simulink model "gate_V37_2016a_HIL_C"  (R2016a) (continued)
== system gate_V37_2016a_HIL_C/Gate/HardwareInputWrapper/MIL_Gate_C == (continued)
  Constant3/1 -> Bus Creator/10  "YellowLedMin"
  Constant5/1 -> Bus Creator/12  "GreenLedMin"
  Constant2/1 -> Bus Creator/9  "YellowLedMax"
  Signal Builder/11(BulbMax) -> Bus Creator/13  "BulbMax"
  Constant1/1 -> Bus Creator/8  "RedLedMin"
  Signal Builder/12(BulbMin) -> Bus Creator/14  "BulbMin"
  Constant6/1 -> Bus Creator/15  "BT_outputMax"
  Constant/1 -> Bus Creator/7  "RedLedMax"
  Signal Builder/10(MCMin) -> Bus Creator/6  "MCMin"
  Signal Builder/9(MCMax) -> Bus Creator/5  "MCMax"
  Signal Builder/8(MRMin) -> Bus Creator/4  "MRMin"
  Signal Builder/7(MRMax) -> Bus Creator/3  "MRMax"
  Signal Builder/6(MLMin) -> Bus Creator/2  "MLMin"
  Signal Builder/5(MLMax) -> Bus Creator/1  "MLMax"
  Constant7/1 -> Bus Creator/16  "BT_outputMin"
  Signal Builder/3(rightSpeedRaw) -> rightCurrentRaw/1
  Signal Builder/4(leftSpeedRaw) -> leftCurrentRaw/1

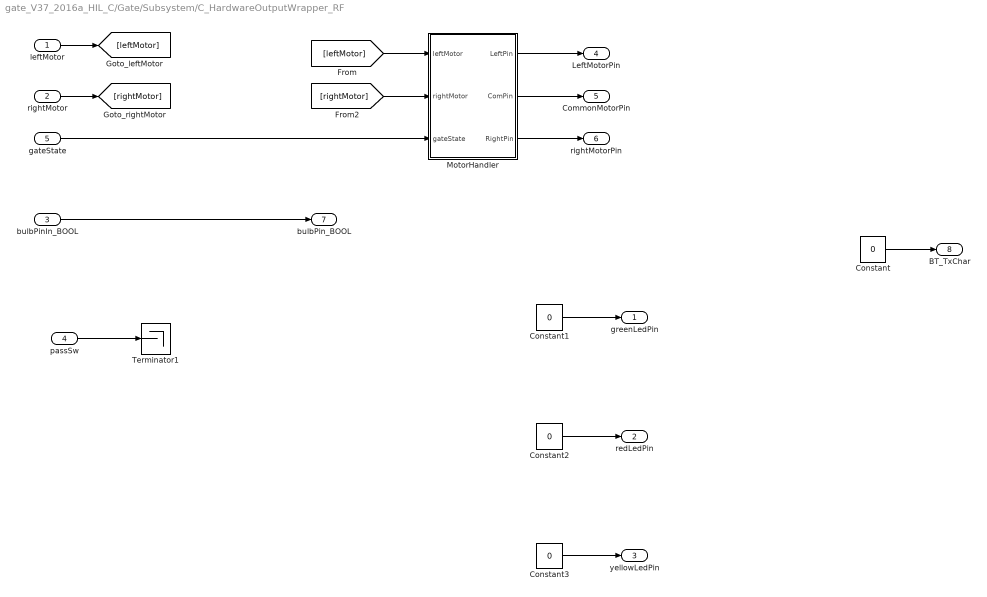
== system gate_V37_2016a_HIL_C/Gate/Subsystem/C_HardwareOutputWrapper_RF ==
  leftMotor : Inport
  rightMotor : Inport  Port=2
  bulbPinIn_BOOL : Inport  Port=3
  passSw : Inport  Port=4
  gateState : Inport  Port=5
  Constant{,1..3} (4 alike) : Constant  Value=0  SampleTime=-1
  MotorHandler : Subsystem
  Terminator1 : Terminator
  greenLedPin : Outport
  redLedPin : Outport  Port=2
  yellowLedPin : Outport  Port=3
  LeftMotorPin : Outport  Port=4
  CommonMotorPin : Outport  Port=5
  rightMotorPin : Outport  Port=6
  bulbPin_BOOL : Outport  Port=7
  BT_TxChar : Outport  Port=8
  wires:
  passSw/1 -> Terminator1/1
  Constant1/1 -> greenLedPin/1
  Constant2/1 -> redLedPin/1
  Constant3/1 -> yellowLedPin/1
  Constant/1 -> BT_TxChar/1
  rightMotor/1 -> [rightMotor]
  leftMotor/1 -> [leftMotor]
  bulbPinIn_BOOL/1 -> bulbPin_BOOL/1
  MotorHandler/3(RightPin) -> rightMotorPin/1
  MotorHandler/2(ComPin) -> CommonMotorPin/1
  MotorHandler/1(LeftPin) -> LeftMotorPin/1
  [rightMotor] -> MotorHandler/2(rightMotor)
  [leftMotor] -> MotorHandler/1(leftMotor)
  gateState/1 -> MotorHandler/3(gateState)

== system gate_V37_2016a_HIL_C/Gate/Subsystem/C_Variant_RF ==
  RFPressedOut_UINT8 : Inport
  passSwitch : Inport  Port=2
  current_sensor : Inport  Port=3
  CLOSED : Constant  Value=CLOSED  SampleTime=-1
  CLOSE_SIDE_VALUE : Constant  Value=CLOSE_SIDE_VALUE  SampleTime=-1
  CLOSING : Constant  Value=CLOSING  SampleTime=-1
  GateLogicStatemachine : Stateflow chart  TreatAsAtomicUnit=on
  INIT : Constant  Value=INIT  SampleTime=-1
  MOVING_TIMER : Constant  Value=MOVING_TIMER  SampleTime=-1
  OPEN : Constant  Value=OPEN  SampleTime=-1
  OPENING : Constant  Value=OPENING  SampleTime=-1
  OPENING_ACTIVE : Constant  Value=OPENING_ACTIVE  SampleTime=-1
  OPEN_SIDE_VALUE : Constant  Value=OPEN_SIDE_VALUE  SampleTime=-1
  STABLE_TIMER_VALUE : Constant  Value=STABLE_TIMER_VALUE  SampleTime=-1
  leftMotor : Outport
  rightMotor : Outport  Port=2
  bulbPin_BOOL : Outport  Port=3
  getState : Outport  Port=4
  wires:
  CLOSING/1 -> GateLogicStatemachine/9(CLOSING)
  OPENING/1 -> GateLogicStatemachine/7(OPENING)
  CLOSED/1 -> GateLogicStatemachine/8(CLOSED)
  OPEN/1 -> GateLogicStatemachine/10(OPEN)
  RFPressedOut_UINT8/1 -> GateLogicStatemachine/1(RFPressedOut_UINT8)
  GateLogicStatemachine/3(bulbPin_BOOL) -> bulbPin_BOOL/1
  current_sensor/1 -> GateLogicStatemachine/3(current_sensor)
  GateLogicStatemachine/1(leftMotor) -> leftMotor/1
  GateLogicStatemachine/2(rightMotor) -> rightMotor/1
  OPENING_ACTIVE/1 -> GateLogicStatemachine/4(OPENING_ACTIVE)
  OPEN_SIDE_VALUE/1 -> GateLogicStatemachine/5(OPEN_SIDE_VALUE)
  CLOSE_SIDE_VALUE/1 -> GateLogicStatemachine/6(CLOSE_SIDE_VALUE)
  passSwitch/1 -> GateLogicStatemachine/2(passSwitch)
  INIT/1 -> GateLogicStatemachine/11(INIT)
  MOVING_TIMER/1 -> GateLogicStatemachine/12(MOVING_TIMER)
  STABLE_TIMER_VALUE/1 -> GateLogicStatemachine/13(STABLE_TIMER_VALUE)
  GateLogicStatemachine/4(gateState) -> getState/1

== system gate_V37_2016a_HIL_C/Gate/Subsystem/CurrentFilter ==
  rightCurrentRaw : Inport
  leftCurrentRaw : Inport  Port=2
  10msSmapler : Stateflow chart  Commented=on  TreatAsAtomicUnit=on
  Subsystem : Subsystem
  current : Outport
  wires:
  leftCurrentRaw/1 -> Subsystem/2(leftCurrentRaw)
  rightCurrentRaw/1 -> Subsystem/1(rightCurrentRaw)
  Subsystem/1(current) -> current/1

== system gate_V37_2016a_HIL_C/Gate/outputPinsBlock/HIL_B ==
  greenLedPin : Inport
  redLedPin : Inport  Port=2
  yellowLed : Inport  Port=3
  leftMotorPin : Inport  Port=4
  commonMotorPin : Inport  Port=5
  rightMotorPin : Inport  Port=6
  bulbPin : Inport  Port=7
  Digital Output : arduinolib/Digital Output  pinNumber=11  blockPlatform=arduino
  Digital Output1 : arduinolib/Digital Output  pinNumber=12  blockPlatform=arduino
  Digital Output2 : arduinolib/Digital Output  pinNumber=13  blockPlatform=arduino
  S-Function Builder1 : S-Function  InitFcn=try, set_param(gcb,'FunctionName','A2PIN_Lib'), end  FunctionName=A2PIN_Lib  SFunctionModules=A2PIN_Lib_wrapper  SFunctionDeploymentMode=off  EnableBusSupport=off  mask(S-Function Builder){}
      mask help: Simulink:masks:SFunctionBuilder_MD
      mask init: same as gate_V37_2016a_HIL_C/Gate/HardwareInputWrapper/HIL_B/S-Function Builder1
      settings: CopyFcn=set_param(gcbh,'LinkStatus','none')  PreSaveFcn=try, set_param(gcb,'FunctionName','A2PIN_Lib'), end  OpenFcn=sfunctionwizard(gcbh)
      mask Display: plot(val(:,1),val(:,2)),disp(sys), port_label('input',1,'input')
  S-Function Builder2 : S-Function  InitFcn=try, set_param(gcb,'FunctionName','A3PIN_Lib'), end  FunctionName=A3PIN_Lib  SFunctionModules=A3PIN_Lib_wrapper  SFunctionDeploymentMode=off  EnableBusSupport=off  mask(S-Function Builder){}
      mask help: Simulink:masks:SFunctionBuilder_MD
      mask init: same as gate_V37_2016a_HIL_C/Gate/HardwareInputWrapper/HIL_B/S-Function Builder1
      settings: CopyFcn=set_param(gcbh,'LinkStatus','none')  PreSaveFcn=try, set_param(gcb,'FunctionName','A3PIN_Lib'), end  OpenFcn=sfunctionwizard(gcbh)
      mask Display: plot(val(:,1),val(:,2)),disp(sys), port_label('input',1,'input')
  S-Function Builder3 : S-Function  InitFcn=try, set_param(gcb,'FunctionName','A4PIN_Lib'), end  FunctionName=A4PIN_Lib  SFunctionModules=A4PIN_Lib_wrapper  SFunctionDeploymentMode=off  EnableBusSupport=off  mask(S-Function Builder){}
      mask help: Simulink:masks:SFunctionBuilder_MD
      mask init: same as gate_V37_2016a_HIL_C/Gate/HardwareInputWrapper/HIL_B/S-Function Builder1
      settings: CopyFcn=set_param(gcbh,'LinkStatus','none')  PreSaveFcn=try, set_param(gcb,'FunctionName','A4PIN_Lib'), end  OpenFcn=sfunctionwizard(gcbh)
      mask Display: plot(val(:,1),val(:,2)),disp(sys), port_label('input',1,'input')
  S-Function Builder4 : S-Function  InitFcn=try, set_param(gcb,'FunctionName','A5PIN_Lib'), end  FunctionName=A5PIN_Lib  SFunctionModules=A5PIN_Lib_wrapper  SFunctionDeploymentMode=off  EnableBusSupport=off  mask(S-Function Builder){}
      mask help: Simulink:masks:SFunctionBuilder_MD
      mask init: same as gate_V37_2016a_HIL_C/Gate/HardwareInputWrapper/HIL_B/S-Function Builder1
      settings: CopyFcn=set_param(gcbh,'LinkStatus','none')  PreSaveFcn=try, set_param(gcb,'FunctionName','A5PIN_Lib'), end  OpenFcn=sfunctionwizard(gcbh)
      mask Display: plot(val(:,1),val(:,2)),disp(sys), port_label('input',1,'input')
  wires:
  yellowLed/1 -> Digital Output2/1
  redLedPin/1 -> Digital Output/1
  greenLedPin/1 -> Digital Output1/1
  commonMotorPin/1 -> S-Function Builder3/1
  leftMotorPin/1 -> S-Function Builder2/1
  rightMotorPin/1 -> S-Function Builder4/1
  bulbPin/1 -> S-Function Builder1/1

== system gate_V37_2016a_HIL_C/Gate/outputPinsBlock/HIL_C ==
  leftMotorPin : Inport
  commonMotorPin : Inport  Port=2
  rightMotorPin : Inport  Port=3
  bulbPin : Inport  Port=4
  S-Function Builder1 : S-Function  InitFcn=try, set_param(gcb,'FunctionName','A2PIN_Lib'), end  FunctionName=A2PIN_Lib  SFunctionModules=A2PIN_Lib_wrapper  SFunctionDeploymentMode=off  EnableBusSupport=off  mask(S-Function Builder){}
      mask help: Simulink:masks:SFunctionBuilder_MD
      mask init: same as gate_V37_2016a_HIL_C/Gate/HardwareInputWrapper/HIL_B/S-Function Builder1
      settings: CopyFcn=set_param(gcbh,'LinkStatus','none')  PreSaveFcn=try, set_param(gcb,'FunctionName','A2PIN_Lib'), end  OpenFcn=sfunctionwizard(gcbh)
      mask Display: plot(val(:,1),val(:,2)),disp(sys), port_label('input',1,'input')
  S-Function Builder2 : S-Function  InitFcn=try, set_param(gcb,'FunctionName','A3PIN_Lib'), end  FunctionName=A3PIN_Lib  SFunctionModules=A3PIN_Lib_wrapper  SFunctionDeploymentMode=off  EnableBusSupport=off  mask(S-Function Builder){}
      mask help: Simulink:masks:SFunctionBuilder_MD
      mask init: same as gate_V37_2016a_HIL_C/Gate/HardwareInputWrapper/HIL_B/S-Function Builder1
      settings: CopyFcn=set_param(gcbh,'LinkStatus','none')  PreSaveFcn=try, set_param(gcb,'FunctionName','A3PIN_Lib'), end  OpenFcn=sfunctionwizard(gcbh)
      mask Display: plot(val(:,1),val(:,2)),disp(sys), port_label('input',1,'input')
  S-Function Builder3 : S-Function  InitFcn=try, set_param(gcb,'FunctionName','A4PIN_Lib'), end  FunctionName=A4PIN_Lib  SFunctionModules=A4PIN_Lib_wrapper  SFunctionDeploymentMode=off  EnableBusSupport=off  mask(S-Function Builder){}
      mask help: Simulink:masks:SFunctionBuilder_MD
      mask init: same as gate_V37_2016a_HIL_C/Gate/HardwareInputWrapper/HIL_B/S-Function Builder1
      settings: CopyFcn=set_param(gcbh,'LinkStatus','none')  PreSaveFcn=try, set_param(gcb,'FunctionName','A4PIN_Lib'), end  OpenFcn=sfunctionwizard(gcbh)
      mask Display: plot(val(:,1),val(:,2)),disp(sys), port_label('input',1,'input')
  S-Function Builder4 : S-Function  InitFcn=try, set_param(gcb,'FunctionName','A5PIN_Lib'), end  FunctionName=A5PIN_Lib  SFunctionModules=A5PIN_Lib_wrapper  SFunctionDeploymentMode=off  EnableBusSupport=off  mask(S-Function Builder){}
      mask help: Simulink:masks:SFunctionBuilder_MD
      mask init: same as gate_V37_2016a_HIL_C/Gate/HardwareInputWrapper/HIL_B/S-Function Builder1
      settings: CopyFcn=set_param(gcbh,'LinkStatus','none')  PreSaveFcn=try, set_param(gcb,'FunctionName','A5PIN_Lib'), end  OpenFcn=sfunctionwizard(gcbh)
      mask Display: plot(val(:,1),val(:,2)),disp(sys), port_label('input',1,'input')
  wires:
  bulbPin/1 -> S-Function Builder1/1
  rightMotorPin/1 -> S-Function Builder4/1
  leftMotorPin/1 -> S-Function Builder2/1
  commonMotorPin/1 -> S-Function Builder3/1

== system gate_V37_2016a_HIL_C/Gate/outputPinsBlock/MIL ==
  "leftMotorPin " : Inport
  "commonMotorPin " : Inport  Port=2
  "rightMotorPin " : Inport  Port=3
  greenLedPin : Inport  Port=4
  redLedPin : Inport  Port=5
  yellowLed : Inport  Port=6
  bulbPin : Inport  Port=7
  BT_TxChar : Inport  Port=8
  SimuOutputLimits : Inport  Port=9
  ScopeBlocks : Subsystem  VariantControl=VisualOutputs
  "VerificationBlocks " : Subsystem  VariantControl=VVOutputs

== system gate_V37_2016a_HIL_C/Gate/HardwareInputWrapper/HIL_B/BluetoothWrapper ==
  S-Function Builder : S-Function  InitFcn=try, set_param(gcb,'FunctionName','BluetoothLib'), end  FunctionName=BluetoothLib  SFunctionModules=BluetoothLib_wrapper  SFunctionDeploymentMode=off  EnableBusSupport=off  mask(S-Function Builder){}
      mask help: Simulink:masks:SFunctionBuilder_MD
      mask init: same as gate_V37_2016a_HIL_C/Gate/HardwareInputWrapper/HIL_B/S-Function Builder1
      settings: CopyFcn=set_param(gcbh,'LinkStatus','none')  PreSaveFcn=try, set_param(gcb,'FunctionName','BluetoothLib'), end  OpenFcn=sfunctionwizard(gcbh)
      mask Display: plot(val(:,1),val(:,2)),disp(sys), port_label('output',1,'BtCharRecieved')
  S-Function Builder1 : S-Function  Commented=on  InitFcn=try, set_param(gcb,'FunctionName','printingBlock'), end  FunctionName=printingBlock  SFunctionModules=printingBlock_wrapper  SFunctionDeploymentMode=off  EnableBusSupport=off  mask(S-Function Builder){}
      mask help: Simulink:masks:SFunctionBuilder_MD
      mask init: same as gate_V37_2016a_HIL_C/Gate/HardwareInputWrapper/HIL_B/S-Function Builder1
      settings: CopyFcn=set_param(gcbh,'LinkStatus','none')  PreSaveFcn=try, set_param(gcb,'FunctionName','printingBlock'), end  OpenFcn=sfunctionwizard(gcbh)
      mask Display: plot(val(:,1),val(:,2)),disp(sys), port_label('input',1,'u0'), port_label('output',1,'y0')
  Unit Delay : UnitDelay  Commented=on  SampleTime=-1
  bluetoothCharPressed : Outport
  wires:
  S-Function Builder/1 -> S-Function Builder1/1, Unit Delay/1, bluetoothCharPressed/1
  Unit Delay/1 -> ?

== system gate_V37_2016a_HIL_C/Gate/HardwareInputWrapper/HIL_B/ButtonsPressed ==
  S-Function Builder1 : S-Function  InitFcn=try, set_param(gcb,'FunctionName','Button_Lib'), end  FunctionName=Button_Lib  SFunctionModules=Button_Lib_wrapper  SFunctionDeploymentMode=off  EnableBusSupport=off  mask(S-Function Builder){}
      mask help: Simulink:masks:SFunctionBuilder_MD
      mask init: same as gate_V37_2016a_HIL_C/Gate/HardwareInputWrapper/HIL_B/S-Function Builder1
      settings: CopyFcn=set_param(gcbh,'LinkStatus','none')  PreSaveFcn=try, set_param(gcb,'FunctionName','Button_Lib'), end  OpenFcn=sfunctionwizard(gcbh)
      mask Display: plot(val(:,1),val(:,2)),disp(sys), port_label('output',1,'RFInput'), port_label('output',2,'passSw')
  RFPressed : Outport
  SWPressed : Outport  Port=2
  wires:
  S-Function Builder1/1 -> RFPressed/1
  S-Function Builder1/2 -> SWPressed/1

== system gate_V37_2016a_HIL_C/Gate/HardwareInputWrapper/HIL_B/EEPROM ==
  passSw : Inport
  passAssigned : Inport  Port=2
  passwordIn : Inport  Port=3
  EEPROM_Control : Stateflow chart  TreatAsAtomicUnit=on
  EEPROM_UPDATE : Subsystem  TreatAsAtomicUnit=on
  Terminator : Terminator
  Unit Delay : UnitDelay  SampleTime=-1
  Unit Delay1 : UnitDelay  SampleTime=-1
  passwordOut : Outport
  passAssignedOut : Outport  Port=2
  wires:
  EEPROM_UPDATE/3(clearComplete) -> Terminator/1
  EEPROM_UPDATE/5(passAssignedOut) -> passAssignedOut/1
  EEPROM_UPDATE/4(passwordOut) -> passwordOut/1
  EEPROM_UPDATE/2(writeComplete) -> [writeComplete]
  [writeComplete] -> Unit Delay1/1
  [readComplete] -> Unit Delay/1
  EEPROM_UPDATE/1(readComplete) -> [readComplete]
  passAssigned/1 -> [passAssigned]
  [passAssigned] -> EEPROM_UPDATE/5(passAssignedIn)
  [passAssigned] -> EEPROM_Control/2(passAssigned)
  passwordIn/1 -> EEPROM_UPDATE/4(passwordIn)
  EEPROM_Control/3(writeReq) -> EEPROM_UPDATE/3(writeReq)
  EEPROM_Control/2(readReq) -> EEPROM_UPDATE/2(readReq)
  EEPROM_Control/1(clearReq) -> EEPROM_UPDATE/1(clearReq)
  Unit Delay1/1 -> EEPROM_Control/4(writeComplete)
  Unit Delay/1 -> EEPROM_Control/3(readComplete)
  passSw/1 -> EEPROM_Control/1(passSw)

== system gate_V37_2016a_HIL_C/Gate/HardwareInputWrapper/HIL_B/KeypadWrapper ==
  KeypadLib : S-Function  InitFcn=try, set_param(gcb,'FunctionName','keypadLib'), end  FunctionName=keypadLib  SFunctionModules=keypadLib_wrapper  SFunctionDeploymentMode=off  EnableBusSupport=off  mask(S-Function Builder){}
      mask help: Simulink:masks:SFunctionBuilder_MD
      mask init: same as gate_V37_2016a_HIL_C/Gate/HardwareInputWrapper/HIL_B/S-Function Builder1
      settings: CopyFcn=set_param(gcbh,'LinkStatus','none')  PreSaveFcn=try, set_param(gcb,'FunctionName','keypadLib'), end  OpenFcn=sfunctionwizard(gcbh)
      mask Display: plot(val(:,1),val(:,2)),disp(sys), port_label('output',1,'charPressed')
  charPressed : Outport
  wires:
  KeypadLib/1 -> charPressed/1

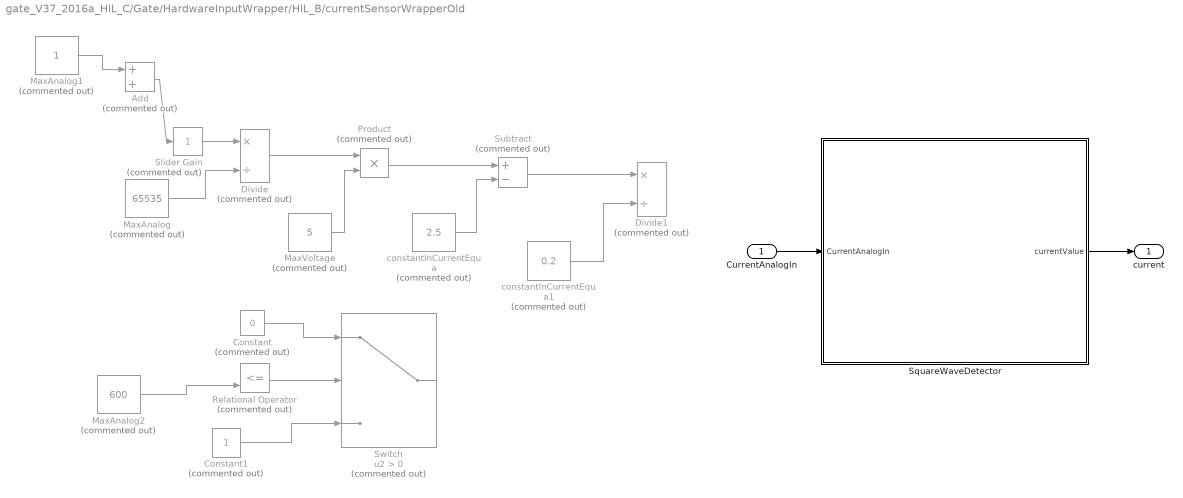
== system gate_V37_2016a_HIL_C/Gate/HardwareInputWrapper/HIL_B/currentSensorWrapperOld ==
  CurrentAnalogIn : Inport
  Add : Sum  Commented=on
  Constant : Constant  Commented=on  Value=0
  Constant1 : Constant  Commented=on
  Divide : Product  Commented=on  Inputs=*/
  Divide1 : Product  Commented=on  Inputs=*/
  MaxAnalog : Constant  Commented=on  Value=65535
  MaxAnalog1 : Constant  Commented=on
  MaxAnalog2 : Constant  Commented=on  Value=600
  MaxVoltage : Constant  Commented=on  Value=5
  Product : Product  Commented=on
  Relational Operator : RelationalOperator  Commented=on  Operator=<=  OutDataTypeStr=boolean
  Slider Gain : simulink/Math Operations/Slider Gain  Commented=on  low=0  gain=1  high=255
  SquareWaveDetector : Stateflow chart  TreatAsAtomicUnit=on
  Subtract : Sum  Commented=on  Inputs=+-
  Switch : Switch  Commented=on  Criteria=u2 > Threshold
  constantInCurrentEqua : Constant  Commented=on  Value=2.5
  constantInCurrentEqua1 : Constant  Commented=on  Value=0.2
  current : Outport
  wires:
  MaxAnalog/1 -> Divide/2
  Divide/1 -> Product/1
  MaxVoltage/1 -> Product/2
  Product/1 -> Subtract/1
  constantInCurrentEqua/1 -> Subtract/2
  Subtract/1 -> Divide1/1
  constantInCurrentEqua1/1 -> Divide1/2
  Slider Gain/1 -> Divide/1
  Add/1 -> Slider Gain/1
  MaxAnalog1/1 -> Add/1
  MaxAnalog2/1 -> Relational Operator/2
  Relational Operator/1 -> Switch/2
  Constant1/1 -> Switch/3
  Constant/1 -> Switch/1
  CurrentAnalogIn/1 -> SquareWaveDetector/1(CurrentAnalogIn)
  SquareWaveDetector/1(currentValue) -> current/1

== system gate_V37_2016a_HIL_C/Gate/HardwareInputWrapper/HIL_C/ButtonsPressed ==
  S-Function Builder1 : S-Function  InitFcn=try, set_param(gcb,'FunctionName','Button_Lib'), end  FunctionName=Button_Lib  SFunctionModules=Button_Lib_wrapper  SFunctionDeploymentMode=off  EnableBusSupport=off  mask(S-Function Builder){}
      mask help: Simulink:masks:SFunctionBuilder_MD
      mask init: same as gate_V37_2016a_HIL_C/Gate/HardwareInputWrapper/HIL_B/S-Function Builder1
      settings: CopyFcn=set_param(gcbh,'LinkStatus','none')  PreSaveFcn=try, set_param(gcb,'FunctionName','Button_Lib'), end  OpenFcn=sfunctionwizard(gcbh)
      mask Display: plot(val(:,1),val(:,2)),disp(sys), port_label('output',1,'RFInput'), port_label('output',2,'passSw')
  RFPressed : Outport
  SWPressed : Outport  Port=2
  wires:
  S-Function Builder1/1 -> RFPressed/1
  S-Function Builder1/2 -> SWPressed/1

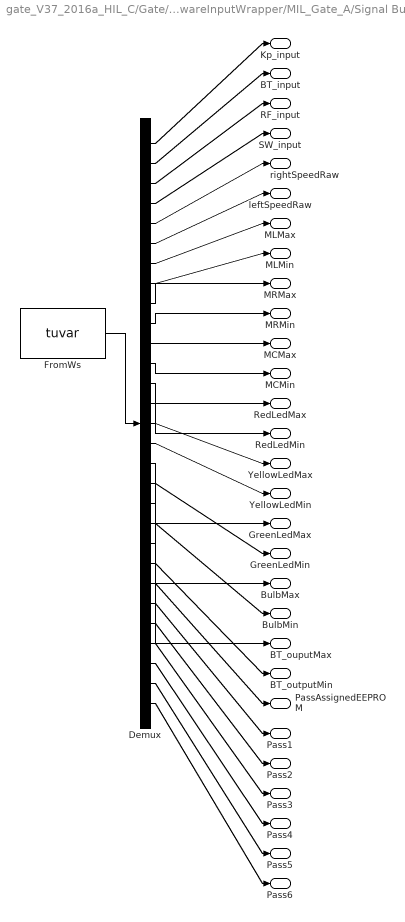
== system gate_V37_2016a_HIL_C/Gate/HardwareInputWrapper/MIL_Gate_A/Signal Builder ==
  Demux : Demux  Tag=STV Demux  Outputs=29
  FromWs : FromWorkspace  Tag=STV FromWs  VariableName=tuvar  SampleTime=0
  Kp_input : Outport  Tag=STV Outport
  BT_input : Outport  Tag=STV Outport  Port=2
  RF_input : Outport  Tag=STV Outport  Port=3
  SW_input : Outport  Tag=STV Outport  Port=4
  rightSpeedRaw : Outport  Tag=STV Outport  Port=5
  leftSpeedRaw : Outport  Tag=STV Outport  Port=6
  MLMax : Outport  Tag=STV Outport  Port=7
  MLMin : Outport  Tag=STV Outport  Port=8
  MRMax : Outport  Tag=STV Outport  Port=9
  MRMin : Outport  Tag=STV Outport  Port=10
  MCMax : Outport  Tag=STV Outport  Port=11
  MCMin : Outport  Tag=STV Outport  Port=12
  RedLedMax : Outport  Tag=STV Outport  Port=13
  RedLedMin : Outport  Tag=STV Outport  Port=14
  YellowLedMax : Outport  Tag=STV Outport  Port=15
  YellowLedMin : Outport  Tag=STV Outport  Port=16
  GreenLedMax : Outport  Tag=STV Outport  Port=17
  GreenLedMin : Outport  Tag=STV Outport  Port=18
  BulbMax : Outport  Tag=STV Outport  Port=19
  BulbMin : Outport  Tag=STV Outport  Port=20
  BT_ouputMax : Outport  Tag=STV Outport  Port=21
  BT_outputMin : Outport  Tag=STV Outport  Port=22
  PassAssignedEEPROM : Outport  Tag=STV Outport  Port=23
  Pass1 : Outport  Tag=STV Outport  Port=24
  Pass2 : Outport  Tag=STV Outport  Port=25
  Pass3 : Outport  Tag=STV Outport  Port=26
  Pass4 : Outport  Tag=STV Outport  Port=27
  Pass5 : Outport  Tag=STV Outport  Port=28
  Pass6 : Outport  Tag=STV Outport  Port=29
  wires:
  FromWs/1 -> Demux/1
  Demux/1 -> Kp_input/1
  Demux/2 -> BT_input/1
  Demux/3 -> RF_input/1
  Demux/4 -> SW_input/1
  Demux/5 -> rightSpeedRaw/1
  Demux/6 -> leftSpeedRaw/1
  Demux/7 -> MLMax/1
  Demux/8 -> MLMin/1
  Demux/9 -> MRMax/1
  Demux/10 -> MRMin/1
  Demux/11 -> MCMax/1
  Demux/12 -> MCMin/1
  Demux/13 -> RedLedMax/1
  Demux/14 -> RedLedMin/1
  Demux/15 -> YellowLedMax/1
  Demux/16 -> YellowLedMin/1
  Demux/17 -> GreenLedMax/1
  Demux/18 -> GreenLedMin/1
  Demux/19 -> BulbMax/1
  Demux/20 -> BulbMin/1
  Demux/21 -> BT_ouputMax/1
  Demux/22 -> BT_outputMin/1
  Demux/23 -> PassAssignedEEPROM/1
  Demux/24 -> Pass1/1
  Demux/25 -> Pass2/1
  Demux/26 -> Pass3/1
  Demux/27 -> Pass4/1
  Demux/28 -> Pass5/1
  Demux/29 -> Pass6/1

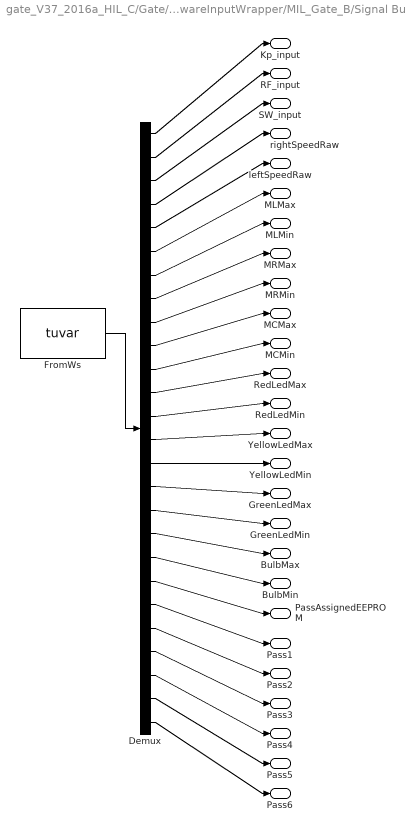
== system gate_V37_2016a_HIL_C/Gate/HardwareInputWrapper/MIL_Gate_B/Signal Builder ==
  Demux : Demux  Tag=STV Demux  Outputs=26
  FromWs : FromWorkspace  Tag=STV FromWs  VariableName=tuvar  SampleTime=0  OutputAfterFinalValue=Holding final value
  Kp_input : Outport  Tag=STV Outport
  RF_input : Outport  Tag=STV Outport  Port=2
  SW_input : Outport  Tag=STV Outport  Port=3
  rightSpeedRaw : Outport  Tag=STV Outport  Port=4
  leftSpeedRaw : Outport  Tag=STV Outport  Port=5
  MLMax : Outport  Tag=STV Outport  Port=6
  MLMin : Outport  Tag=STV Outport  Port=7
  MRMax : Outport  Tag=STV Outport  Port=8
  MRMin : Outport  Tag=STV Outport  Port=9
  MCMax : Outport  Tag=STV Outport  Port=10
  MCMin : Outport  Tag=STV Outport  Port=11
  RedLedMax : Outport  Tag=STV Outport  Port=12
  RedLedMin : Outport  Tag=STV Outport  Port=13
  YellowLedMax : Outport  Tag=STV Outport  Port=14
  YellowLedMin : Outport  Tag=STV Outport  Port=15
  GreenLedMax : Outport  Tag=STV Outport  Port=16
  GreenLedMin : Outport  Tag=STV Outport  Port=17
  BulbMax : Outport  Tag=STV Outport  Port=18
  BulbMin : Outport  Tag=STV Outport  Port=19
  PassAssignedEEPROM : Outport  Tag=STV Outport  Port=20
  Pass1 : Outport  Tag=STV Outport  Port=21
  Pass2 : Outport  Tag=STV Outport  Port=22
  Pass3 : Outport  Tag=STV Outport  Port=23
  Pass4 : Outport  Tag=STV Outport  Port=24
  Pass5 : Outport  Tag=STV Outport  Port=25
  Pass6 : Outport  Tag=STV Outport  Port=26
  wires:
  FromWs/1 -> Demux/1
  Demux/1 -> Kp_input/1
  Demux/2 -> RF_input/1
  Demux/3 -> SW_input/1
  Demux/4 -> rightSpeedRaw/1
  Demux/5 -> leftSpeedRaw/1
  Demux/6 -> MLMax/1
  Demux/7 -> MLMin/1
  Demux/8 -> MRMax/1
  Demux/9 -> MRMin/1
  Demux/10 -> MCMax/1
  Demux/11 -> MCMin/1
  Demux/12 -> RedLedMax/1
  Demux/13 -> RedLedMin/1
  Demux/14 -> YellowLedMax/1
  Demux/15 -> YellowLedMin/1
  Demux/16 -> GreenLedMax/1
  Demux/17 -> GreenLedMin/1
  Demux/18 -> BulbMax/1
  Demux/19 -> BulbMin/1
  Demux/20 -> PassAssignedEEPROM/1
  Demux/21 -> Pass1/1
  Demux/22 -> Pass2/1
  Demux/23 -> Pass3/1
  Demux/24 -> Pass4/1
  Demux/25 -> Pass5/1
  Demux/26 -> Pass6/1

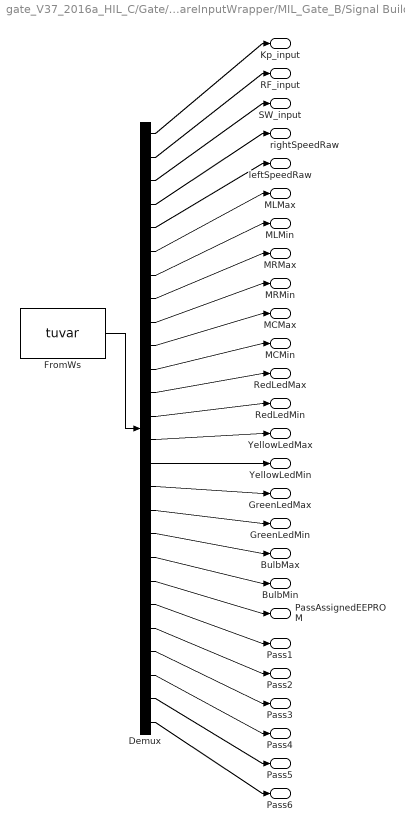
== system gate_V37_2016a_HIL_C/Gate/HardwareInputWrapper/MIL_Gate_B/Signal Builder1 ==
  Demux : Demux  Tag=STV Demux  Outputs=26
  FromWs : FromWorkspace  Tag=STV FromWs  VariableName=tuvar  SampleTime=0  OutputAfterFinalValue=Holding final value
  Kp_input : Outport  Tag=STV Outport
  RF_input : Outport  Tag=STV Outport  Port=2
  SW_input : Outport  Tag=STV Outport  Port=3
  rightSpeedRaw : Outport  Tag=STV Outport  Port=4
  leftSpeedRaw : Outport  Tag=STV Outport  Port=5
  MLMax : Outport  Tag=STV Outport  Port=6
  MLMin : Outport  Tag=STV Outport  Port=7
  MRMax : Outport  Tag=STV Outport  Port=8
  MRMin : Outport  Tag=STV Outport  Port=9
  MCMax : Outport  Tag=STV Outport  Port=10
  MCMin : Outport  Tag=STV Outport  Port=11
  RedLedMax : Outport  Tag=STV Outport  Port=12
  RedLedMin : Outport  Tag=STV Outport  Port=13
  YellowLedMax : Outport  Tag=STV Outport  Port=14
  YellowLedMin : Outport  Tag=STV Outport  Port=15
  GreenLedMax : Outport  Tag=STV Outport  Port=16
  GreenLedMin : Outport  Tag=STV Outport  Port=17
  BulbMax : Outport  Tag=STV Outport  Port=18
  BulbMin : Outport  Tag=STV Outport  Port=19
  PassAssignedEEPROM : Outport  Tag=STV Outport  Port=20
  Pass1 : Outport  Tag=STV Outport  Port=21
  Pass2 : Outport  Tag=STV Outport  Port=22
  Pass3 : Outport  Tag=STV Outport  Port=23
  Pass4 : Outport  Tag=STV Outport  Port=24
  Pass5 : Outport  Tag=STV Outport  Port=25
  Pass6 : Outport  Tag=STV Outport  Port=26
  wires:
  Demux/26 -> Pass6/1
  Demux/25 -> Pass5/1
  Demux/24 -> Pass4/1
  Demux/23 -> Pass3/1
  Demux/22 -> Pass2/1
  Demux/21 -> Pass1/1
  Demux/20 -> PassAssignedEEPROM/1
  Demux/19 -> BulbMin/1
  Demux/18 -> BulbMax/1
  Demux/17 -> GreenLedMin/1
  Demux/16 -> GreenLedMax/1
  Demux/15 -> YellowLedMin/1
  Demux/14 -> YellowLedMax/1
  Demux/13 -> RedLedMin/1
  Demux/12 -> RedLedMax/1
  Demux/11 -> MCMin/1
  Demux/10 -> MCMax/1
  Demux/9 -> MRMin/1
  Demux/8 -> MRMax/1
  Demux/7 -> MLMin/1
  Demux/6 -> MLMax/1
  Demux/5 -> leftSpeedRaw/1
  Demux/4 -> rightSpeedRaw/1
  Demux/3 -> SW_input/1
  Demux/2 -> RF_input/1
  Demux/1 -> Kp_input/1
  FromWs/1 -> Demux/1

== system gate_V37_2016a_HIL_C/Gate/HardwareInputWrapper/MIL_Gate_C/Signal Builder ==
  Demux : Demux  Tag=STV Demux  Outputs=12
  FromWs : FromWorkspace  Tag=STV FromWs  VariableName=tuvar  SampleTime=0
      SigBuilderData=|
      | common.afterFinalStr = 'Extrapolate'
      | common.sampleTime = 0
      | dataSet(1).timeRange = [0 120]
      | dataSet(1).name = 'RFinputOnly_C'
      | dataSet(2).timeRange = [0 120]
      | dataSet(2).name = 'RF_SW_input_C'
      | dataSet(3).timeRange = [0 120]
      | dataSet(3).name = 'SW_PauseBeforeSideDelay_C'
      | dataSet(4).timeRange = [0 120]
      | dataSet(4).name = 'SW_input2Cycles_C'
      | dataSet(5).timeRange = [0 120]
      | dataSet(5).name = 'RF_PauseBeforeSideDelay_C'
      | dataSet(6).timeRange = [0 120]
      | dataSet(6).name = 'SW_PauseBeforeSideDelayClose_C'
      | sbobj.Groups(1).Name = 'RFinputOnly_C'
      | sbobj.Groups(1).Signals(1).Name = 'RF_input'
      | sbobj.Groups(1).Signals(1).XData = [0 3.85 3.85 4.01 4.01 31.15 31.15 31.347 31.347 120]
      | sbobj.Groups(1).Signals(1).YData = [0 0 1 1 0 0 1 1 0 0]
      | sbobj.Groups(1).Signals(2).Name = 'SW_input'
      | sbobj.Groups(1).Signals(2).XData = [0 120]
      | sbobj.Groups(1).Signals(2).YData = [0 0]
      | sbobj.Groups(1).Signals(3).Name = 'rightSpeedRaw'
      | sbobj.Groups(1).Signals(3).XData = [0 0.001 0.002 0.003 0.004 0.005 0.006 0.007 0.008 0.009 0.01 0.011 ... (60001 elements, 1x60001)]
      | sbobj.Groups(1).Signals(3).YData = [1010 1010 1010 1010 1010 1010 1010 1010 1010 1010 1010 1010 ... (60001 elements, 1x60001)]
      | sbobj.Groups(1).Signals(4).Name = 'leftSpeedRaw'
      | sbobj.Groups(1).Signals(4).XData = [0 48 48 72 72 120]
      | sbobj.Groups(1).Signals(4).YData = [0 0 1 1 0 0]
      | sbobj.Groups(1).Signals(5).Name = 'MLMax'
      | sbobj.Groups(1).Signals(5).XData = [0 3.5 3.5 19.5 19.5 31 31 35 35 120]
      | sbobj.Groups(1).Signals(5).YData = [0.1 0.1 1.1 1.1 0.1 0.1 1.1 1.1 0.1 0.1]
      | sbobj.Groups(1).Signals(6).Name = 'MLMin'
      | sbobj.Groups(1).Signals(6).XData = [0 4.05 4.05 17.6 17.6 31.5 31.5 33.7 33.7 120]
      | sbobj.Groups(1).Signals(6).YData = [-0.1 -0.1 0.9 0.9 -0.1 -0.1 0.9 0.9 -0.1 -0.1]
      | sbobj.Groups(1).Signals(7).Name = 'MRMax'
      | sbobj.Groups(1).Signals(7).XData = [0 6.75 6.75 19.5 19.5 30.35 120]
      | sbobj.Groups(1).Signals(7).YData = [0.1 0.1 1.1 1.1 0.1 0.1 0.1]
      | sbobj.Groups(1).Signals(8).Name = 'MRMin'
      | sbobj.Groups(1).Signals(8).XData = [0 8.1 8.1 18.5 18.5 120]
      | sbobj.Groups(1).Signals(8).YData = [-0.1 -0.1 0.9 0.9 -0.1 -0.1]
      | sbobj.Groups(1).Signals(9).Name = 'MCMax'
      | sbobj.Groups(1).Signals(9).XData = [0 3.49 3.49 31.9 31.9 46.2 120]
      | sbobj.Groups(1).Signals(9).YData = [0.1 0.1 1.1 1.1 0.1 0.1 0.1]
      | sbobj.Groups(1).Signals(10).Name = 'MCMin'
      | sbobj.Groups(1).Signals(10).XData = [0 4.01 4.01 31 31 47.7 120]
      | sbobj.Groups(1).Signals(10).YData = [-0.1 -0.1 0.9 0.9 -0.1 -0.1 -0.1]
      | sbobj.Groups(1).Signals(11).Name = 'BulbMax'
      | sbobj.Groups(1).Signals(11).XData = [0 3.76 3.76 48.3 48.3 120]
      | sbobj.Groups(1).Signals(11).YData = [0.1 0.1 1.1 1.1 0.1 0.1]
      | sbobj.Groups(1).Signals(12).Name = 'BulbMin'
      | sbobj.Groups(1).Signals(12).XData = [0 4.27 4.27 45.5 45.5 120]
      | sbobj.Groups(1).Signals(12).YData = [-0.1 -0.1 0.9 0.9 -0.1 -0.1]
      | sbobj.Groups(1).TimeRange = [0 120]
      | sbobj.Groups(2).Name = 'RF_SW_input_C'
      | sbobj.Groups(2).Signals(1).Name = 'RF_input'
      | sbobj.Groups(2).Signals(1).XData = [0 3.85 3.85 4.01 4.01 120]
      | sbobj.Groups(2).Signals(1).YData = [0 0 1 1 0 0]
      | sbobj.Groups(2).Signals(2).Name = 'SW_input'
      | sbobj.Groups(2).Signals(2).XData = [0 31.17 31.17 31.65 31.65 120]
      | sbobj.Groups(2).Signals(2).YData = [0 0 1 1 0 0]
      | sbobj.Groups(2).Signals(3).Name = 'rightSpeedRaw'
      | sbobj.Groups(2).Signals(3).XData = [0 0.001 0.002 0.003 0.004 0.005 0.006 0.007 0.008 0.009 0.01 0.011 ... (60001 elements, 1x60001)]
      | sbobj.Groups(2).Signals(3).YData = [1010 1010 1010 1010 1010 1010 1010 1010 1010 1010 1010 1010 ... (60001 elements, 1x60001)]
      | sbobj.Groups(2).Signals(4).Name = 'leftSpeedRaw'
      | sbobj.Groups(2).Signals(4).XData = [0 48 48 72 72 120]
      | sbobj.Groups(2).Signals(4).YData = [0 0 1 1 0 0]
      | sbobj.Groups(2).Signals(5).Name = 'MLMax'
      | sbobj.Groups(2).Signals(5).XData = [0 3.5 3.5 19.5 19.5 31 31 35 35 120]
      | sbobj.Groups(2).Signals(5).YData = [0.1 0.1 1.1 1.1 0.1 0.1 1.1 1.1 0.1 0.1]
      | sbobj.Groups(2).Signals(6).Name = 'MLMin'
      | sbobj.Groups(2).Signals(6).XData = [0 4.05 4.05 18.5 18.5 31.5 31.5 33.9 33.9 120]
      | sbobj.Groups(2).Signals(6).YData = [-0.1 -0.1 0.9 0.9 -0.1 -0.1 0.9 0.9 -0.1 -0.1]
      | sbobj.Groups(2).Signals(7).Name = 'MRMax'
      | sbobj.Groups(2).Signals(7).XData = [0 6.75 6.75 19.5 19.5 30.35 120]
      | sbobj.Groups(2).Signals(7).YData = [0.1 0.1 1.1 1.1 0.1 0.1 0.1]
      | sbobj.Groups(2).Signals(8).Name = 'MRMin'
      | sbobj.Groups(2).Signals(8).XData = [0 8.3 8.3 17.8 17.8 120]
      | sbobj.Groups(2).Signals(8).YData = [-0.1 -0.1 0.9 0.9 -0.1 -0.1]
      | sbobj.Groups(2).Signals(9).Name = 'MCMax'
      | sbobj.Groups(2).Signals(9).XData = [0 3.51 3.51 31.9 31.9 46.2 120]
      | sbobj.Groups(2).Signals(9).YData = [0.1 0.1 1.1 1.1 0.1 0.1 0.1]
      | sbobj.Groups(2).Signals(10).Name = 'MCMin'
      | sbobj.Groups(2).Signals(10).XData = [0 4.17 4.17 31 31 47.7 120]
      | sbobj.Groups(2).Signals(10).YData = [-0.1 -0.1 0.9 0.9 -0.1 -0.1 -0.1]
      | sbobj.Groups(2).Signals(11).Name = 'BulbMax'
      | sbobj.Groups(2).Signals(11).XData = [0 3.76 3.76 47.7 47.7 120]
      | sbobj.Groups(2).Signals(11).YData = [0.1 0.1 1.1 1.1 0.1 0.1]
      | sbobj.Groups(2).Signals(12).Name = 'BulbMin'
      | sbobj.Groups(2).Signals(12).XData = [0 4.27 4.27 46.2 46.2 120]
      | sbobj.Groups(2).Signals(12).YData = [-0.1 -0.1 0.9 0.9 -0.1 -0.1]
      | sbobj.Groups(2).TimeRange = [0 120]
      | sbobj.Groups(3).Name = 'SW_PauseBeforeSideDelay_C'
      | sbobj.Groups(3).Signals(1).Name = 'RF_input'
      | sbobj.Groups(3).Signals(1).XData = [0 120]
      | sbobj.Groups(3).Signals(1).YData = [0 0]
      | sbobj.Groups(3).Signals(2).Name = 'SW_input'
      | sbobj.Groups(3).Signals(2).XData = [0 3.88 3.88 4.55 4.55 5.5 5.5 6 6 11.35 11.35 12.1 12.1 31.17 31.17 31.65 31.65 120]
      | sbobj.Groups(3).Signals(2).YData = [0 0 1 1 0 0 1 1 0 0 1 1 0 0 1 1 0 0]
      | sbobj.Groups(3).Signals(3).Name = 'rightSpeedRaw'
      | sbobj.Groups(3).Signals(3).XData = [0 0.001 0.002 0.003 0.004 0.005 0.006 0.007 0.008 0.009 0.01 0.011 ... (60001 elements, 1x60001)]
      | sbobj.Groups(3).Signals(3).YData = [1010 1010 1010 1010 1010 1010 1010 1010 1010 1010 1010 1010 ... (60001 elements, 1x60001)]
      | sbobj.Groups(3).Signals(4).Name = 'leftSpeedRaw'
      | sbobj.Groups(3).Signals(4).XData = [0 0.001 0.002 0.003 0.004 0.005 0.006 0.007 0.008 0.009 0.01 0.011 ... (60001 elements, 1x60001)]
      | sbobj.Groups(3).Signals(4).YData = [1010 1010 1010 1010 1010 1010 1010 1010 1010 1010 1010 1010 ... (60001 elements, 1x60001)]
      | sbobj.Groups(3).Signals(5).Name = 'MLMax'
      | sbobj.Groups(3).Signals(5).XData = [0 3.5 3.5 12.5 12.5 31 31 35 35 120]
      | sbobj.Groups(3).Signals(5).YData = [0.1 0.1 1.1 1.1 0.1 0.1 1.1 1.1 0.1 0.1]
      | sbobj.Groups(3).Signals(6).Name = 'MLMin'
      | sbobj.Groups(3).Signals(6).XData = [0 4.05 4.05 10.6 10.6 31.5 31.5 33.8 33.8 120]
      | sbobj.Groups(3).Signals(6).YData = [-0.1 -0.1 0.9 0.9 -0.1 -0.1 0.9 0.9 -0.1 -0.1]
      | sbobj.Groups(3).Signals(7).Name = 'MRMax'
      | sbobj.Groups(3).Signals(7).XData = [0 6.75 6.75 12.9 12.9 30.35 120]
      | sbobj.Groups(3).Signals(7).YData = [0.1 0.1 1.1 1.1 0.1 0.1 0.1]
      | sbobj.Groups(3).Signals(8).Name = 'MRMin'
      | sbobj.Groups(3).Signals(8).XData = [0 8.3 8.3 10.4 10.4 120]
      | sbobj.Groups(3).Signals(8).YData = [-0.1 -0.1 0.9 0.9 -0.1 -0.1]
      | sbobj.Groups(3).Signals(9).Name = 'MCMax'
      | sbobj.Groups(3).Signals(9).XData = [0 3.535 3.535 31.9 31.9 46.2 120]
      | sbobj.Groups(3).Signals(9).YData = [0.1 0.1 1.1 1.1 0.1 0.1 0.1]
      | sbobj.Groups(3).Signals(10).Name = 'MCMin'
      | sbobj.Groups(3).Signals(10).XData = [0 4.37 4.37 31 31 47.7 120]
      | sbobj.Groups(3).Signals(10).YData = [-0.1 -0.1 0.9 0.9 -0.1 -0.1 -0.1]
      | sbobj.Groups(3).Signals(11).Name = 'BulbMax'
      | sbobj.Groups(3).Signals(11).XData = [0 3.76 3.76 47.7 47.7 120]
      | sbobj.Groups(3).Signals(11).YData = [0.1 0.1 1.1 1.1 0.1 0.1]
      | sbobj.Groups(3).Signals(12).Name = 'BulbMin'
      | sbobj.Groups(3).Signals(12).XData = [0 4.27 4.27 46.2 46.2 120]
      | sbobj.Groups(3).Signals(12).YData = [-0.1 -0.1 0.9 0.9 -0.1 -0.1]
      | sbobj.Groups(3).TimeRange = [0 120]
      | sbobj.Groups(4).Name = 'SW_input2Cycles_C'
      | sbobj.Groups(4).Signals(1).Name = 'RF_input'
      | sbobj.Groups(4).Signals(1).XData = [0 23 23 23.2 23.2 120]
      | sbobj.Groups(4).Signals(1).YData = [0 0 1 1 0 0]
      | sbobj.Groups(4).Signals(2).Name = 'SW_input'
      | sbobj.Groups(4).Signals(2).XData = [0 3.85 3.85 4.1 4.1 43 43 43.88 43.88 79 79 80.14 80.14 120]
      | sbobj.Groups(4).Signals(2).YData = [0 0 1 1 0 0 1 1 0 0 1 1 0 0]
      | sbobj.Groups(4).Signals(3).Name = 'rightSpeedRaw'
      | sbobj.Groups(4).Signals(3).XData = [0 0.001 0.002 0.003 0.004 0.005 0.006 0.007 0.008 0.009 0.01 0.011 ... (120001 elements, 1x120001)]
      | sbobj.Groups(4).Signals(3).YData = [1010 1010 1010 1010 1010 1010 1010 1010 1010 1010 1010 1010 ... (120001 elements, 1x120001)]
      | sbobj.Groups(4).Signals(4).Name = 'leftSpeedRaw'
      | sbobj.Groups(4).Signals(4).XData = [0 0.001 0.002 0.003 0.004 0.005 0.006 0.007 0.008 0.009 0.01 0.011 ... (119980 elements, 1x119980)]
      | sbobj.Groups(4).Signals(4).YData = [1010 1010 1010 1010 1010 1010 1010 1010 1010 1010 1010 1010 ... (119980 elements, 1x119980)]
      | sbobj.Groups(4).Signals(5).Name = 'MLMax'
      | sbobj.Groups(4).Signals(5).XData = [0 3.5 3.5 19.5 19.5 22.5 22.5 26.5 26.5 42.5 42.5 58.5 58.5 78.5 78.5 82.5 82.5 120]
      | sbobj.Groups(4).Signals(5).YData = [0.1 0.1 1.1 1.1 0.1 0.1 1.1 1.1 0.1 0.1 1.1 1.1 0.1 0.1 1.1 1.1 0.1 0.1]
      | sbobj.Groups(4).Signals(6).Name = 'MLMin'
      | sbobj.Groups(4).Signals(6).XData = [0 4.05 4.05 18.5 18.5 23.5 23.5 25.5 25.5 43.5 43.5 57.5 57.5 79.5 79.5 81.5 81.5 120]
      | sbobj.Groups(4).Signals(6).YData = [-0.1 -0.1 0.9 0.9 -0.1 -0.1 0.9 0.9 -0.1 -0.1 0.9 0.9 -0.1 -0.1 0.9 0.9 -0.1 -0.1]
      | sbobj.Groups(4).Signals(7).Name = 'MRMax'
      | sbobj.Groups(4).Signals(7).XData = [0 6.75 6.75 19.5 19.5 45.5 45.5 58.5 58.5 120]
      | sbobj.Groups(4).Signals(7).YData = [0.1 0.1 1.1 1.1 0.1 0.1 1.1 1.1 0.1 0.1]
      | sbobj.Groups(4).Signals(8).Name = 'MRMin'
      | sbobj.Groups(4).Signals(8).XData = [0 8.6 8.6 18.5 18.5 47.4 47.4 57.5 57.5 120]
      | sbobj.Groups(4).Signals(8).YData = [-0.1 -0.1 0.9 0.9 -0.1 -0.1 0.9 0.9 -0.1 -0.1]
      | sbobj.Groups(4).Signals(9).Name = 'MCMax'
      | sbobj.Groups(4).Signals(9).XData = [0 3.445 3.445 23.5 23.5 37.5 42.24 42.24 79.5 79.5 93.5 120]
      | sbobj.Groups(4).Signals(9).YData = [0.1 0.1 1.1 1.1 0.1 0.1 0.1 1.1 1.1 0.1 0.1 0.1]
      | sbobj.Groups(4).Signals(10).Name = 'MCMin'
      | sbobj.Groups(4).Signals(10).XData = [0 4.285 4.285 22.5 22.5 38.5 44.27 44.27 78.5 78.5 94.5 120]
      | sbobj.Groups(4).Signals(10).YData = [-0.1 -0.1 0.9 0.9 -0.1 -0.1 -0.1 0.9 0.9 -0.1 -0.1 -0.1]
      | sbobj.Groups(4).Signals(11).Name = 'BulbMax'
      | sbobj.Groups(4).Signals(11).XData = [0 3.76 3.76 38.9 38.9 42.5 42.5 94.5 94.5 120]
      | sbobj.Groups(4).Signals(11).YData = [0.1 0.1 1.1 1.1 0.1 0.1 1.1 1.1 0.1 0.1]
      | sbobj.Groups(4).Signals(12).Name = 'BulbMin'
      | sbobj.Groups(4).Signals(12).XData = [0 4.27 4.27 37.9 37.9 43.5 43.5 93.5 93.5 120]
      | sbobj.Groups(4).Signals(12).YData = [-0.1 -0.1 0.9 0.9 -0.1 -0.1 0.9 0.9 -0.1 -0.1]
      | sbobj.Groups(4).TimeRange = [0 120]
      | sbobj.Groups(5).Name = 'RF_PauseBeforeSideDelay_C'
      | sbobj.Groups(5).Signals(1).Name = 'RF_input'
      | sbobj.Groups(5).Signals(1).XData = [0 3.88 3.88 4.55 4.55 5.5 5.5 6 6 11.35 11.35 12.1 12.1 31.17 31.17 31.65 31.65 32.3 32.3 32.7 32.7 40.85 40.85 41.2 41.2 120]
      | sbobj.Groups(5).Signals(1).YData = [0 0 1 1 0 0 1 1 0 0 1 1 0 0 1 1 0 0 1 1 0 0 1 1 0 0]
      | sbobj.Groups(5).Signals(2).Name = 'SW_input'
      | sbobj.Groups(5).Signals(2).XData = [0 120]
      | sbobj.Groups(5).Signals(2).YData = [0 0]
      | sbobj.Groups(5).Signals(3).Name = 'rightSpeedRaw'
      | sbobj.Groups(5).Signals(3).XData = [0 0.001 0.002 0.003 0.004 0.005 0.006 0.007 0.008 0.009 0.01 0.011 ... (60001 elements, 1x60001)]
      | sbobj.Groups(5).Signals(3).YData = [1010 1010 1010 1010 1010 1010 1010 1010 1010 1010 1010 1010 ... (60001 elements, 1x60001)]
      | sbobj.Groups(5).Signals(4).Name = 'leftSpeedRaw'
      | sbobj.Groups(5).Signals(4).XData = [0 0.001 0.002 0.003 0.004 0.005 0.006 0.007 0.008 0.009 0.01 0.011 ... (60001 elements, 1x60001)]
      | sbobj.Groups(5).Signals(4).YData = [1010 1010 1010 1010 1010 1010 1010 1010 1010 1010 1010 1010 ... (60001 elements, 1x60001)]
      | sbobj.Groups(5).Signals(5).Name = 'MLMax'
      | sbobj.Groups(5).Signals(5).XData = [0 3.5 3.5 12.5 12.5 31 31 35 35 120]
      | sbobj.Groups(5).Signals(5).YData = [0.1 0.1 1.1 1.1 0.1 0.1 1.1 1.1 0.1 0.1]
      | sbobj.Groups(5).Signals(6).Name = 'MLMin'
      | sbobj.Groups(5).Signals(6).XData = [0 4.05 4.05 10.6 10.6 31.5 31.5 33.8 33.8 120]
      | sbobj.Groups(5).Signals(6).YData = [-0.1 -0.1 0.9 0.9 -0.1 -0.1 0.9 0.9 -0.1 -0.1]
      | sbobj.Groups(5).Signals(7).Name = 'MRMax'
      | sbobj.Groups(5).Signals(7).XData = [0 6.75 6.75 12.9 12.9 30.35 120]
      | sbobj.Groups(5).Signals(7).YData = [0.1 0.1 1.1 1.1 0.1 0.1 0.1]
      | sbobj.Groups(5).Signals(8).Name = 'MRMin'
      | sbobj.Groups(5).Signals(8).XData = [0 8.3 8.3 10.4 10.4 120]
      | sbobj.Groups(5).Signals(8).YData = [-0.1 -0.1 0.9 0.9 -0.1 -0.1]
      | sbobj.Groups(5).Signals(9).Name = 'MCMax'
      | sbobj.Groups(5).Signals(9).XData = [0 3.535 3.535 31.9 31.9 46.2 120]
      | sbobj.Groups(5).Signals(9).YData = [0.1 0.1 1.1 1.1 0.1 0.1 0.1]
      | sbobj.Groups(5).Signals(10).Name = 'MCMin'
      | sbobj.Groups(5).Signals(10).XData = [0 4.37 4.37 31 31 47.7 120]
      | sbobj.Groups(5).Signals(10).YData = [-0.1 -0.1 0.9 0.9 -0.1 -0.1 -0.1]
      | sbobj.Groups(5).Signals(11).Name = 'BulbMax'
      | sbobj.Groups(5).Signals(11).XData = [0 3.76 3.76 42.25 42.25 120]
      | sbobj.Groups(5).Signals(11).YData = [0.1 0.1 1.1 1.1 0.1 0.1]
      | sbobj.Groups(5).Signals(12).Name = 'BulbMin'
      | sbobj.Groups(5).Signals(12).XData = [0 4.27 4.27 40 40 120]
      | sbobj.Groups(5).Signals(12).YData = [-0.1 -0.1 0.9 0.9 -0.1 -0.1]
      | sbobj.Groups(5).TimeRange = [0 120]
      | sbobj.Groups(6).Name = 'SW_PauseBeforeSideDelayClose_C'
      | sbobj.Groups(6).Signals(1).Name = 'RF_input'
      | sbobj.Groups(6).Signals(1).XData = [0 3.88 120]
      | sbobj.Groups(6).Signals(1).YData = [0 0 0]
      | sbobj.Groups(6).Signals(2).Name = 'SW_input'
      | sbobj.Groups(6).Signals(2).XData = [0 3.88 3.88 4.55 4.55 5.5 5.5 6 6 11.35 11.35 12.1 12.1 31.17 31.17 31.65 31.65 32.3 32.3 32.7 32.7 40.85 40.85 41.2 41.2 120]
      | sbobj.Groups(6).Signals(2).YData = [0 0 1 1 0 0 1 1 0 0 1 1 0 0 1 1 0 0 1 1 0 0 1 1 0 0]
      | sbobj.Groups(6).Signals(3).Name = 'rightSpeedRaw'
      | sbobj.Groups(6).Signals(3).XData = [0 0.001 0.002 0.003 0.004 0.005 0.006 0.007 0.008 0.009 0.01 0.011 ... (60001 elements, 1x60001)]
      | sbobj.Groups(6).Signals(3).YData = [1010 1010 1010 1010 1010 1010 1010 1010 1010 1010 1010 1010 ... (60001 elements, 1x60001)]
      | sbobj.Groups(6).Signals(4).Name = 'leftSpeedRaw'
      | sbobj.Groups(6).Signals(4).XData = [0 0.001 0.002 0.003 0.004 0.005 0.006 0.007 0.008 0.009 0.01 0.011 ... (60001 elements, 1x60001)]
      | sbobj.Groups(6).Signals(4).YData = [1010 1010 1010 1010 1010 1010 1010 1010 1010 1010 1010 1010 ... (60001 elements, 1x60001)]
      | sbobj.Groups(6).Signals(5).Name = 'MLMax'
      | sbobj.Groups(6).Signals(5).XData = [0 3.5 3.5 12.5 12.5 31 31 35 35 120]
      | sbobj.Groups(6).Signals(5).YData = [0.1 0.1 1.1 1.1 0.1 0.1 1.1 1.1 0.1 0.1]
      | sbobj.Groups(6).Signals(6).Name = 'MLMin'
      | sbobj.Groups(6).Signals(6).XData = [0 4.05 4.05 10.6 10.6 31.5 31.5 33.8 33.8 120]
      | sbobj.Groups(6).Signals(6).YData = [-0.1 -0.1 0.9 0.9 -0.1 -0.1 0.9 0.9 -0.1 -0.1]
      | sbobj.Groups(6).Signals(7).Name = 'MRMax'
      | sbobj.Groups(6).Signals(7).XData = [0 6.75 6.75 12.9 12.9 30.35 120]
      | sbobj.Groups(6).Signals(7).YData = [0.1 0.1 1.1 1.1 0.1 0.1 0.1]
      | sbobj.Groups(6).Signals(8).Name = 'MRMin'
      | sbobj.Groups(6).Signals(8).XData = [0 8.3 8.3 10.4 10.4 120]
      | sbobj.Groups(6).Signals(8).YData = [-0.1 -0.1 0.9 0.9 -0.1 -0.1]
      | sbobj.Groups(6).Signals(9).Name = 'MCMax'
      | sbobj.Groups(6).Signals(9).XData = [0 3.535 3.535 31.9 31.9 46.2 120]
      | sbobj.Groups(6).Signals(9).YData = [0.1 0.1 1.1 1.1 0.1 0.1 0.1]
      | sbobj.Groups(6).Signals(10).Name = 'MCMin'
      | sbobj.Groups(6).Signals(10).XData = [0 4.37 4.37 31 31 47.7 120]
      | sbobj.Groups(6).Signals(10).YData = [-0.1 -0.1 0.9 0.9 -0.1 -0.1 -0.1]
      | sbobj.Groups(6).Signals(11).Name = 'BulbMax'
      | sbobj.Groups(6).Signals(11).XData = [0 3.76 3.76 42.25 42.25 120]
      | sbobj.Groups(6).Signals(11).YData = [0.1 0.1 1.1 1.1 0.1 0.1]
      | sbobj.Groups(6).Signals(12).Name = 'BulbMin'
      | sbobj.Groups(6).Signals(12).XData = [0 4.27 4.27 40 40 120]
      | sbobj.Groups(6).Signals(12).YData = [-0.1 -0.1 0.9 0.9 -0.1 -0.1]
      | sbobj.Groups(6).TimeRange = [0 120]
  RF_input : Outport  Tag=STV Outport
  SW_input : Outport  Tag=STV Outport  Port=2
  rightSpeedRaw : Outport  Tag=STV Outport  Port=3
  leftSpeedRaw : Outport  Tag=STV Outport  Port=4
  MLMax : Outport  Tag=STV Outport  Port=5
  MLMin : Outport  Tag=STV Outport  Port=6
  MRMax : Outport  Tag=STV Outport  Port=7
  MRMin : Outport  Tag=STV Outport  Port=8
  MCMax : Outport  Tag=STV Outport  Port=9
  MCMin : Outport  Tag=STV Outport  Port=10
  BulbMax : Outport  Tag=STV Outport  Port=11
  BulbMin : Outport  Tag=STV Outport  Port=12
  wires:
  FromWs/1 -> Demux/1
  Demux/1 -> RF_input/1
  Demux/2 -> SW_input/1
  Demux/3 -> rightSpeedRaw/1
  Demux/4 -> leftSpeedRaw/1
  Demux/5 -> MLMax/1
  Demux/6 -> MLMin/1
  Demux/7 -> MRMax/1
  Demux/8 -> MRMin/1
  Demux/9 -> MCMax/1
  Demux/10 -> MCMin/1
  Demux/11 -> BulbMax/1
  Demux/12 -> BulbMin/1
